simulink model "bee2_calib_corr"  (Simulink 7.1)
settings: Solver=ode45  StartTime=0.0  StopTime=100  RelTol=1e-3
undefined symbols (not defined in the file or in its folder): BEE2_usr
libraries: xbsIndex_r4 x2291, quant_xcorr_lib x1792, xps_library x56, xbsReplacements_r4 x32, casper_library_misc x2
(part 11 of 30)

== system bee2_calib_corr/slice6 ==
  in : Inport  OutDataType=sfix(16)  OutScaling=2^0
  Slice1 : xbsIndex_r4/Slice  nbits=2  boolean_output=off  mode=Upper Bit Location + Width  bit1=-2  base1=MSB of Input  bit0=0  base0=LSB of Input  dbl_ovrd=off  block_type=slice
      settings: infoedit="Extracts a given range of bits from each input sample and presents it at the output.  The output type is ordinarily unsigned with binary point at zero, but can be Boolean when the slice is one bit wide.<P><P>Hardware notes: In hardware this block costs nothing."  block_version=10.1.3
  Slice2 : xbsIndex_r4/Slice  nbits=2  boolean_output=off  mode=Upper Bit Location + Width  bit1=-6  base1=MSB of Input  bit0=0  base0=LSB of Input  dbl_ovrd=off  block_type=slice
      settings: infoedit="Extracts a given range of bits from each input sample and presents it at the output.  The output type is ordinarily unsigned with binary point at zero, but can be Boolean when the slice is one bit wide.<P><P>Hardware notes: In hardware this block costs nothing."  block_version=10.1.3
  Slice26 : xbsIndex_r4/Slice  nbits=2  boolean_output=off  mode=Upper Bit Location + Width  bit1=0  base1=MSB of Input  bit0=0  base0=LSB of Input  dbl_ovrd=off  block_type=slice
      settings: infoedit="Extracts a given range of bits from each input sample and presents it at the output.  The output type is ordinarily unsigned with binary point at zero, but can be Boolean when the slice is one bit wide.<P><P>Hardware notes: In hardware this block costs nothing."  block_version=10.1.3
  Slice3 : xbsIndex_r4/Slice  nbits=2  boolean_output=off  mode=Upper Bit Location + Width  bit1=-4  base1=MSB of Input  bit0=0  base0=LSB of Input  dbl_ovrd=off  block_type=slice
      settings: infoedit="Extracts a given range of bits from each input sample and presents it at the output.  The output type is ordinarily unsigned with binary point at zero, but can be Boolean when the slice is one bit wide.<P><P>Hardware notes: In hardware this block costs nothing."  block_version=10.1.3
  Slice4 : xbsIndex_r4/Slice  nbits=2  boolean_output=off  mode=Upper Bit Location + Width  bit1=-10  base1=MSB of Input  bit0=0  base0=LSB of Input  dbl_ovrd=off  block_type=slice
      settings: infoedit="Extracts a given range of bits from each input sample and presents it at the output.  The output type is ordinarily unsigned with binary point at zero, but can be Boolean when the slice is one bit wide.<P><P>Hardware notes: In hardware this block costs nothing."  block_version=10.1.3
  Slice5 : xbsIndex_r4/Slice  nbits=2  boolean_output=off  mode=Upper Bit Location + Width  bit1=-14  base1=MSB of Input  bit0=0  base0=LSB of Input  dbl_ovrd=off  block_type=slice
      settings: infoedit="Extracts a given range of bits from each input sample and presents it at the output.  The output type is ordinarily unsigned with binary point at zero, but can be Boolean when the slice is one bit wide.<P><P>Hardware notes: In hardware this block costs nothing."  block_version=10.1.3
  Slice6 : xbsIndex_r4/Slice  nbits=2  boolean_output=off  mode=Upper Bit Location + Width  bit1=-8  base1=MSB of Input  bit0=0  base0=LSB of Input  dbl_ovrd=off  block_type=slice
      settings: infoedit="Extracts a given range of bits from each input sample and presents it at the output.  The output type is ordinarily unsigned with binary point at zero, but can be Boolean when the slice is one bit wide.<P><P>Hardware notes: In hardware this block costs nothing."  block_version=10.1.3
  Slice7 : xbsIndex_r4/Slice  nbits=2  boolean_output=off  mode=Upper Bit Location + Width  bit1=-12  base1=MSB of Input  bit0=0  base0=LSB of Input  dbl_ovrd=off  block_type=slice
      settings: infoedit="Extracts a given range of bits from each input sample and presents it at the output.  The output type is ordinarily unsigned with binary point at zero, but can be Boolean when the slice is one bit wide.<P><P>Hardware notes: In hardware this block costs nothing."  block_version=10.1.3
  out0 : Outport  OutDataType=sfix(16)  OutScaling=2^0
  out1 : Outport  Port=2  OutDataType=sfix(16)  OutScaling=2^0
  out2 : Outport  Port=3  OutDataType=sfix(16)  OutScaling=2^0
  out3 : Outport  Port=4  OutDataType=sfix(16)  OutScaling=2^0
  out4 : Outport  Port=5  OutDataType=sfix(16)  OutScaling=2^0
  out5 : Outport  Port=6  OutDataType=sfix(16)  OutScaling=2^0
  out6 : Outport  Port=7  OutDataType=sfix(16)  OutScaling=2^0
  out7 : Outport  Port=8  OutDataType=sfix(16)  OutScaling=2^0
  wires:
  in/1 -> Slice26/1, Slice5/1, Slice7/1, Slice4/1, Slice6/1, Slice2/1, Slice3/1, Slice1/1
  Slice26/1 -> out0/1
  Slice1/1 -> out1/1
  Slice3/1 -> out2/1
  Slice2/1 -> out3/1
  Slice6/1 -> out4/1
  Slice4/1 -> out5/1
  Slice7/1 -> out6/1
  Slice5/1 -> out7/1

== system bee2_calib_corr/slice7 ==
  in : Inport  OutDataType=sfix(16)  OutScaling=2^0
  Slice1 : xbsIndex_r4/Slice  nbits=2  boolean_output=off  mode=Upper Bit Location + Width  bit1=-2  base1=MSB of Input  bit0=0  base0=LSB of Input  dbl_ovrd=off  block_type=slice
      settings: infoedit="Extracts a given range of bits from each input sample and presents it at the output.  The output type is ordinarily unsigned with binary point at zero, but can be Boolean when the slice is one bit wide.<P><P>Hardware notes: In hardware this block costs nothing."  block_version=10.1.3
  Slice2 : xbsIndex_r4/Slice  nbits=2  boolean_output=off  mode=Upper Bit Location + Width  bit1=-6  base1=MSB of Input  bit0=0  base0=LSB of Input  dbl_ovrd=off  block_type=slice
      settings: infoedit="Extracts a given range of bits from each input sample and presents it at the output.  The output type is ordinarily unsigned with binary point at zero, but can be Boolean when the slice is one bit wide.<P><P>Hardware notes: In hardware this block costs nothing."  block_version=10.1.3
  Slice26 : xbsIndex_r4/Slice  nbits=2  boolean_output=off  mode=Upper Bit Location + Width  bit1=0  base1=MSB of Input  bit0=0  base0=LSB of Input  dbl_ovrd=off  block_type=slice
      settings: infoedit="Extracts a given range of bits from each input sample and presents it at the output.  The output type is ordinarily unsigned with binary point at zero, but can be Boolean when the slice is one bit wide.<P><P>Hardware notes: In hardware this block costs nothing."  block_version=10.1.3
  Slice3 : xbsIndex_r4/Slice  nbits=2  boolean_output=off  mode=Upper Bit Location + Width  bit1=-4  base1=MSB of Input  bit0=0  base0=LSB of Input  dbl_ovrd=off  block_type=slice
      settings: infoedit="Extracts a given range of bits from each input sample and presents it at the output.  The output type is ordinarily unsigned with binary point at zero, but can be Boolean when the slice is one bit wide.<P><P>Hardware notes: In hardware this block costs nothing."  block_version=10.1.3
  Slice4 : xbsIndex_r4/Slice  nbits=2  boolean_output=off  mode=Upper Bit Location + Width  bit1=-10  base1=MSB of Input  bit0=0  base0=LSB of Input  dbl_ovrd=off  block_type=slice
      settings: infoedit="Extracts a given range of bits from each input sample and presents it at the output.  The output type is ordinarily unsigned with binary point at zero, but can be Boolean when the slice is one bit wide.<P><P>Hardware notes: In hardware this block costs nothing."  block_version=10.1.3
  Slice5 : xbsIndex_r4/Slice  nbits=2  boolean_output=off  mode=Upper Bit Location + Width  bit1=-14  base1=MSB of Input  bit0=0  base0=LSB of Input  dbl_ovrd=off  block_type=slice
      settings: infoedit="Extracts a given range of bits from each input sample and presents it at the output.  The output type is ordinarily unsigned with binary point at zero, but can be Boolean when the slice is one bit wide.<P><P>Hardware notes: In hardware this block costs nothing."  block_version=10.1.3
  Slice6 : xbsIndex_r4/Slice  nbits=2  boolean_output=off  mode=Upper Bit Location + Width  bit1=-8  base1=MSB of Input  bit0=0  base0=LSB of Input  dbl_ovrd=off  block_type=slice
      settings: infoedit="Extracts a given range of bits from each input sample and presents it at the output.  The output type is ordinarily unsigned with binary point at zero, but can be Boolean when the slice is one bit wide.<P><P>Hardware notes: In hardware this block costs nothing."  block_version=10.1.3
  Slice7 : xbsIndex_r4/Slice  nbits=2  boolean_output=off  mode=Upper Bit Location + Width  bit1=-12  base1=MSB of Input  bit0=0  base0=LSB of Input  dbl_ovrd=off  block_type=slice
      settings: infoedit="Extracts a given range of bits from each input sample and presents it at the output.  The output type is ordinarily unsigned with binary point at zero, but can be Boolean when the slice is one bit wide.<P><P>Hardware notes: In hardware this block costs nothing."  block_version=10.1.3
  out0 : Outport  OutDataType=sfix(16)  OutScaling=2^0
  out1 : Outport  Port=2  OutDataType=sfix(16)  OutScaling=2^0
  out2 : Outport  Port=3  OutDataType=sfix(16)  OutScaling=2^0
  out3 : Outport  Port=4  OutDataType=sfix(16)  OutScaling=2^0
  out4 : Outport  Port=5  OutDataType=sfix(16)  OutScaling=2^0
  out5 : Outport  Port=6  OutDataType=sfix(16)  OutScaling=2^0
  out6 : Outport  Port=7  OutDataType=sfix(16)  OutScaling=2^0
  out7 : Outport  Port=8  OutDataType=sfix(16)  OutScaling=2^0
  wires:
  Slice5/1 -> out7/1
  Slice7/1 -> out6/1
  Slice4/1 -> out5/1
  Slice6/1 -> out4/1
  Slice2/1 -> out3/1
  Slice3/1 -> out2/1
  Slice1/1 -> out1/1
  Slice26/1 -> out0/1
  in/1 -> Slice1/1, Slice3/1, Slice2/1, Slice6/1, Slice4/1, Slice7/1, Slice5/1, Slice26/1

== system bee2_calib_corr/slice8 ==
  in : Inport  OutDataType=sfix(16)  OutScaling=2^0
  Slice1 : xbsIndex_r4/Slice  nbits=2  boolean_output=off  mode=Upper Bit Location + Width  bit1=-2  base1=MSB of Input  bit0=0  base0=LSB of Input  dbl_ovrd=off  block_type=slice
      settings: infoedit="Extracts a given range of bits from each input sample and presents it at the output.  The output type is ordinarily unsigned with binary point at zero, but can be Boolean when the slice is one bit wide.<P><P>Hardware notes: In hardware this block costs nothing."  block_version=10.1.3
  Slice2 : xbsIndex_r4/Slice  nbits=2  boolean_output=off  mode=Upper Bit Location + Width  bit1=-6  base1=MSB of Input  bit0=0  base0=LSB of Input  dbl_ovrd=off  block_type=slice
      settings: infoedit="Extracts a given range of bits from each input sample and presents it at the output.  The output type is ordinarily unsigned with binary point at zero, but can be Boolean when the slice is one bit wide.<P><P>Hardware notes: In hardware this block costs nothing."  block_version=10.1.3
  Slice26 : xbsIndex_r4/Slice  nbits=2  boolean_output=off  mode=Upper Bit Location + Width  bit1=0  base1=MSB of Input  bit0=0  base0=LSB of Input  dbl_ovrd=off  block_type=slice
      settings: infoedit="Extracts a given range of bits from each input sample and presents it at the output.  The output type is ordinarily unsigned with binary point at zero, but can be Boolean when the slice is one bit wide.<P><P>Hardware notes: In hardware this block costs nothing."  block_version=10.1.3
  Slice3 : xbsIndex_r4/Slice  nbits=2  boolean_output=off  mode=Upper Bit Location + Width  bit1=-4  base1=MSB of Input  bit0=0  base0=LSB of Input  dbl_ovrd=off  block_type=slice
      settings: infoedit="Extracts a given range of bits from each input sample and presents it at the output.  The output type is ordinarily unsigned with binary point at zero, but can be Boolean when the slice is one bit wide.<P><P>Hardware notes: In hardware this block costs nothing."  block_version=10.1.3
  Slice4 : xbsIndex_r4/Slice  nbits=2  boolean_output=off  mode=Upper Bit Location + Width  bit1=-10  base1=MSB of Input  bit0=0  base0=LSB of Input  dbl_ovrd=off  block_type=slice
      settings: infoedit="Extracts a given range of bits from each input sample and presents it at the output.  The output type is ordinarily unsigned with binary point at zero, but can be Boolean when the slice is one bit wide.<P><P>Hardware notes: In hardware this block costs nothing."  block_version=10.1.3
  Slice5 : xbsIndex_r4/Slice  nbits=2  boolean_output=off  mode=Upper Bit Location + Width  bit1=-14  base1=MSB of Input  bit0=0  base0=LSB of Input  dbl_ovrd=off  block_type=slice
      settings: infoedit="Extracts a given range of bits from each input sample and presents it at the output.  The output type is ordinarily unsigned with binary point at zero, but can be Boolean when the slice is one bit wide.<P><P>Hardware notes: In hardware this block costs nothing."  block_version=10.1.3
  Slice6 : xbsIndex_r4/Slice  nbits=2  boolean_output=off  mode=Upper Bit Location + Width  bit1=-8  base1=MSB of Input  bit0=0  base0=LSB of Input  dbl_ovrd=off  block_type=slice
      settings: infoedit="Extracts a given range of bits from each input sample and presents it at the output.  The output type is ordinarily unsigned with binary point at zero, but can be Boolean when the slice is one bit wide.<P><P>Hardware notes: In hardware this block costs nothing."  block_version=10.1.3
  Slice7 : xbsIndex_r4/Slice  nbits=2  boolean_output=off  mode=Upper Bit Location + Width  bit1=-12  base1=MSB of Input  bit0=0  base0=LSB of Input  dbl_ovrd=off  block_type=slice
      settings: infoedit="Extracts a given range of bits from each input sample and presents it at the output.  The output type is ordinarily unsigned with binary point at zero, but can be Boolean when the slice is one bit wide.<P><P>Hardware notes: In hardware this block costs nothing."  block_version=10.1.3
  out0 : Outport  OutDataType=sfix(16)  OutScaling=2^0
  out1 : Outport  Port=2  OutDataType=sfix(16)  OutScaling=2^0
  out2 : Outport  Port=3  OutDataType=sfix(16)  OutScaling=2^0
  out3 : Outport  Port=4  OutDataType=sfix(16)  OutScaling=2^0
  out4 : Outport  Port=5  OutDataType=sfix(16)  OutScaling=2^0
  out5 : Outport  Port=6  OutDataType=sfix(16)  OutScaling=2^0
  out6 : Outport  Port=7  OutDataType=sfix(16)  OutScaling=2^0
  out7 : Outport  Port=8  OutDataType=sfix(16)  OutScaling=2^0
  wires:
  in/1 -> Slice26/1, Slice5/1, Slice7/1, Slice4/1, Slice6/1, Slice2/1, Slice3/1, Slice1/1
  Slice26/1 -> out0/1
  Slice1/1 -> out1/1
  Slice3/1 -> out2/1
  Slice2/1 -> out3/1
  Slice6/1 -> out4/1
  Slice4/1 -> out5/1
  Slice7/1 -> out6/1
  Slice5/1 -> out7/1

== system bee2_calib_corr/slice9 ==
  in : Inport  OutDataType=sfix(16)  OutScaling=2^0
  Slice1 : xbsIndex_r4/Slice  nbits=2  boolean_output=off  mode=Upper Bit Location + Width  bit1=-2  base1=MSB of Input  bit0=0  base0=LSB of Input  dbl_ovrd=off  block_type=slice
      settings: infoedit="Extracts a given range of bits from each input sample and presents it at the output.  The output type is ordinarily unsigned with binary point at zero, but can be Boolean when the slice is one bit wide.<P><P>Hardware notes: In hardware this block costs nothing."  block_version=10.1.3
  Slice2 : xbsIndex_r4/Slice  nbits=2  boolean_output=off  mode=Upper Bit Location + Width  bit1=-6  base1=MSB of Input  bit0=0  base0=LSB of Input  dbl_ovrd=off  block_type=slice
      settings: infoedit="Extracts a given range of bits from each input sample and presents it at the output.  The output type is ordinarily unsigned with binary point at zero, but can be Boolean when the slice is one bit wide.<P><P>Hardware notes: In hardware this block costs nothing."  block_version=10.1.3
  Slice26 : xbsIndex_r4/Slice  nbits=2  boolean_output=off  mode=Upper Bit Location + Width  bit1=0  base1=MSB of Input  bit0=0  base0=LSB of Input  dbl_ovrd=off  block_type=slice
      settings: infoedit="Extracts a given range of bits from each input sample and presents it at the output.  The output type is ordinarily unsigned with binary point at zero, but can be Boolean when the slice is one bit wide.<P><P>Hardware notes: In hardware this block costs nothing."  block_version=10.1.3
  Slice3 : xbsIndex_r4/Slice  nbits=2  boolean_output=off  mode=Upper Bit Location + Width  bit1=-4  base1=MSB of Input  bit0=0  base0=LSB of Input  dbl_ovrd=off  block_type=slice
      settings: infoedit="Extracts a given range of bits from each input sample and presents it at the output.  The output type is ordinarily unsigned with binary point at zero, but can be Boolean when the slice is one bit wide.<P><P>Hardware notes: In hardware this block costs nothing."  block_version=10.1.3
  Slice4 : xbsIndex_r4/Slice  nbits=2  boolean_output=off  mode=Upper Bit Location + Width  bit1=-10  base1=MSB of Input  bit0=0  base0=LSB of Input  dbl_ovrd=off  block_type=slice
      settings: infoedit="Extracts a given range of bits from each input sample and presents it at the output.  The output type is ordinarily unsigned with binary point at zero, but can be Boolean when the slice is one bit wide.<P><P>Hardware notes: In hardware this block costs nothing."  block_version=10.1.3
  Slice5 : xbsIndex_r4/Slice  nbits=2  boolean_output=off  mode=Upper Bit Location + Width  bit1=-14  base1=MSB of Input  bit0=0  base0=LSB of Input  dbl_ovrd=off  block_type=slice
      settings: infoedit="Extracts a given range of bits from each input sample and presents it at the output.  The output type is ordinarily unsigned with binary point at zero, but can be Boolean when the slice is one bit wide.<P><P>Hardware notes: In hardware this block costs nothing."  block_version=10.1.3
  Slice6 : xbsIndex_r4/Slice  nbits=2  boolean_output=off  mode=Upper Bit Location + Width  bit1=-8  base1=MSB of Input  bit0=0  base0=LSB of Input  dbl_ovrd=off  block_type=slice
      settings: infoedit="Extracts a given range of bits from each input sample and presents it at the output.  The output type is ordinarily unsigned with binary point at zero, but can be Boolean when the slice is one bit wide.<P><P>Hardware notes: In hardware this block costs nothing."  block_version=10.1.3
  Slice7 : xbsIndex_r4/Slice  nbits=2  boolean_output=off  mode=Upper Bit Location + Width  bit1=-12  base1=MSB of Input  bit0=0  base0=LSB of Input  dbl_ovrd=off  block_type=slice
      settings: infoedit="Extracts a given range of bits from each input sample and presents it at the output.  The output type is ordinarily unsigned with binary point at zero, but can be Boolean when the slice is one bit wide.<P><P>Hardware notes: In hardware this block costs nothing."  block_version=10.1.3
  out0 : Outport  OutDataType=sfix(16)  OutScaling=2^0
  out1 : Outport  Port=2  OutDataType=sfix(16)  OutScaling=2^0
  out2 : Outport  Port=3  OutDataType=sfix(16)  OutScaling=2^0
  out3 : Outport  Port=4  OutDataType=sfix(16)  OutScaling=2^0
  out4 : Outport  Port=5  OutDataType=sfix(16)  OutScaling=2^0
  out5 : Outport  Port=6  OutDataType=sfix(16)  OutScaling=2^0
  out6 : Outport  Port=7  OutDataType=sfix(16)  OutScaling=2^0
  out7 : Outport  Port=8  OutDataType=sfix(16)  OutScaling=2^0
  wires:
  Slice5/1 -> out7/1
  Slice7/1 -> out6/1
  Slice4/1 -> out5/1
  Slice6/1 -> out4/1
  Slice2/1 -> out3/1
  Slice3/1 -> out2/1
  Slice1/1 -> out1/1
  Slice26/1 -> out0/1
  in/1 -> Slice1/1, Slice3/1, Slice2/1, Slice6/1, Slice4/1, Slice7/1, Slice5/1, Slice26/1

== system bee2_calib_corr/valid ==
  ld0 : Inport  OutDataType=sfix(16)  OutScaling=2^0
  ld1 : Inport  Port=2  OutDataType=sfix(16)  OutScaling=2^0
  align : Inport  Port=3  OutDataType=sfix(16)  OutScaling=2^0
  Constant1 : xbsIndex_r4/Constant  arith_type=Boolean  const=1  n_bits=1  bin_pt=0  explicit_period=on  period=1  equ=P=C  opselect=C  inp2=PCIN>>17  opr=+  inp1=P  carry=CIN  dbl_ovrd=off  block_type=constant
  Constant3 : xbsIndex_r4/Constant  arith_type=Boolean  const=1  n_bits=1  bin_pt=0  explicit_period=on  period=1  equ=P=C  opselect=C  inp2=PCIN>>17  opr=+  inp1=P  carry=CIN  dbl_ovrd=off  block_type=constant
  Convert1 : xbsIndex_r4/Convert  arith_type=Boolean  n_bits=8  bin_pt=6  quantization=Truncate  overflow=Wrap  latency=0  dbl_ovrd=off  pipeline=off  block_type=convert
      settings: xl_area=[0, 0, 0, 0, 0, 0, 0]  block_version=10.1.3
  Convert3 : xbsIndex_r4/Convert  arith_type=Boolean  n_bits=8  bin_pt=6  quantization=Truncate  overflow=Wrap  latency=0  dbl_ovrd=off  pipeline=off  block_type=convert
      settings: xl_area=[0, 0, 0, 0, 0, 0, 0]  block_version=10.1.3
  Expression : xbsIndex_r4/Expression  expression=a & b & c  align_bp=on  en=off  latency=0  precision=Full  arith_type=Unsigned  n_bits=8  bin_pt=2  dbl_ovrd=off  block_type=expr
      settings: xl_area=[0, 0, 0, 0, 0, 0, 0]  block_version=10.1.3
  Inverter1 : xbsIndex_r4/Inverter  en=off  latency=0  dbl_ovrd=off  block_type=inv
      settings: xl_area=[0, 0, 0, 0, 0, 0, 0]
  Inverter2 : xbsIndex_r4/Inverter  en=off  latency=0  dbl_ovrd=off  block_type=inv
      settings: xl_area=[0, 0, 0, 0, 0, 0, 0]
  Simulation Multiplexer1 : xbsIndex_r4/Simulation Multiplexer  sim_sel=1  hw_sel=2  block_type=simmux
      settings: infoedit="Distinguish input subsystems as \"simulation only\" and \"generation only\".  The input specified For Simulation will be used during Simulink simulation.  The input specified For Generation will be used during code generation.  This block will typically be used on the output of a HW Co-Simulation, Black Box, or ModelSim subsystem.<P><P>Hardware Notes: This block costs nothing."  block_version=10.1.3
  Simulation Multiplexer3 : xbsIndex_r4/Simulation Multiplexer  sim_sel=1  hw_sel=2  block_type=simmux
      settings: infoedit="Distinguish input subsystems as \"simulation only\" and \"generation only\".  The input specified For Simulation will be used during Simulink simulation.  The input specified For Generation will be used during code generation.  This block will typically be used on the output of a HW Co-Simulation, Black Box, or ModelSim subsystem.<P><P>Hardware Notes: This block costs nothing."  block_version=10.1.3
  get : Outport  OutDataType=sfix(16)  OutScaling=2^0
  wires:
  Convert1/1 -> Inverter1/1
  Convert3/1 -> Inverter2/1
  ld0/1 -> Convert1/1
  ld1/1 -> Convert3/1
  align/1 -> Expression/3
  Expression/1 -> get/1
  Constant1/1 -> Simulation Multiplexer1/1
  Inverter1/1 -> Simulation Multiplexer1/2
  Constant3/1 -> Simulation Multiplexer3/1
  Inverter2/1 -> Simulation Multiplexer3/2
  Simulation Multiplexer3/1 -> Expression/2
  Simulation Multiplexer1/1 -> Expression/1

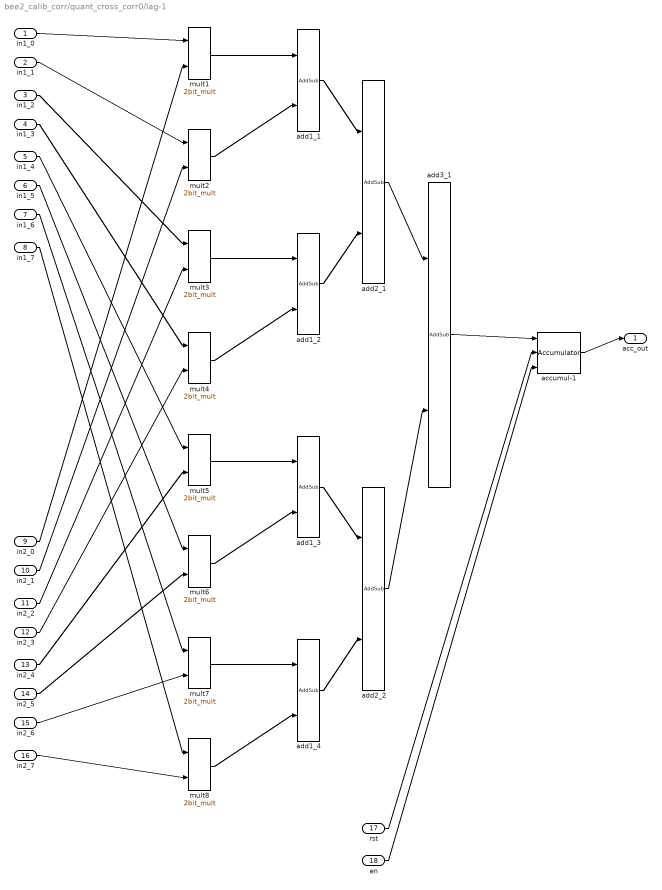
== system bee2_calib_corr/quant_cross_corr0/lag-1 ==
  in1_0 : Inport  OutDataType=sfix(16)  OutScaling=2^0
  in1_1 : Inport  Port=2  OutDataType=sfix(16)  OutScaling=2^0
  in1_2 : Inport  Port=3  OutDataType=sfix(16)  OutScaling=2^0
  in1_3 : Inport  Port=4  OutDataType=sfix(16)  OutScaling=2^0
  in1_4 : Inport  Port=5  OutDataType=sfix(16)  OutScaling=2^0
  in1_5 : Inport  Port=6  OutDataType=sfix(16)  OutScaling=2^0
  in1_6 : Inport  Port=7  OutDataType=sfix(16)  OutScaling=2^0
  in1_7 : Inport  Port=8  OutDataType=sfix(16)  OutScaling=2^0
  in2_0 : Inport  Port=9  OutDataType=sfix(16)  OutScaling=2^0
  in2_1 : Inport  Port=10  OutDataType=sfix(16)  OutScaling=2^0
  in2_2 : Inport  Port=11  OutDataType=sfix(16)  OutScaling=2^0
  in2_3 : Inport  Port=12  OutDataType=sfix(16)  OutScaling=2^0
  in2_4 : Inport  Port=13  OutDataType=sfix(16)  OutScaling=2^0
  in2_5 : Inport  Port=14  OutDataType=sfix(16)  OutScaling=2^0
  in2_6 : Inport  Port=15  OutDataType=sfix(16)  OutScaling=2^0
  in2_7 : Inport  Port=16  OutDataType=sfix(16)  OutScaling=2^0
  rst : Inport  Port=17  OutDataType=sfix(16)  OutScaling=2^0
  en : Inport  Port=18  OutDataType=sfix(16)  OutScaling=2^0
  accumul-1 : xbsIndex_r4/Accumulator  operation=Add  n_bits=32  overflow=Saturate  scale=1  rst=on  hasbypass=off  en=on  dbl_ovrd=off  use_behavioral_HDL=on  implementation=Fabric  block_type=accum
      settings: infoedit="Adder or subtractor-based accumulator.   Output type and binary point position match the input.<P><P>Hardware notes: When \"Reinitialize with input 'b' on reset\" is selected, the accumulator is forced to run at the system rate even if the input 'b' is running at a slower rate."  xl_area=[0, 0, 0, 0, 0, 0, 0]  block_version=10.1.3
  add1_{1..4} (4 alike) : xbsIndex_r4/AddSub  mode=Addition  use_carryin=off  use_carryout=off  en=off  latency=1  precision=Full  arith_type=Unsigned  n_bits=8  bin_pt=2  quantization=Truncate  overflow=Wrap  dbl_ovrd=off  use_behavioral_HDL=on  pipelined=on  use_rpm=on  hw_selection=Fabric  block_type=addsub
      settings: xl_area=[0, 0, 0, 0, 0, 0, 0]  block_version=10.1.3
  add2_1 : xbsIndex_r4/AddSub  mode=Addition  use_carryin=off  use_carryout=off  en=off  latency=1  precision=Full  arith_type=Unsigned  n_bits=8  bin_pt=2  quantization=Truncate  overflow=Wrap  dbl_ovrd=off  use_behavioral_HDL=on  pipelined=on  use_rpm=on  hw_selection=Fabric  block_type=addsub
      settings: xl_area=[0, 0, 0, 0, 0, 0, 0]  block_version=10.1.3
  add2_2 : xbsIndex_r4/AddSub  mode=Addition  use_carryin=off  use_carryout=off  en=off  latency=1  precision=Full  arith_type=Unsigned  n_bits=8  bin_pt=2  quantization=Truncate  overflow=Wrap  dbl_ovrd=off  use_behavioral_HDL=on  pipelined=on  use_rpm=on  hw_selection=Fabric  block_type=addsub
      settings: xl_area=[0, 0, 0, 0, 0, 0, 0]  block_version=10.1.3
  add3_1 : xbsIndex_r4/AddSub  mode=Addition  use_carryin=off  use_carryout=off  en=off  latency=1  precision=Full  arith_type=Unsigned  n_bits=8  bin_pt=2  quantization=Truncate  overflow=Wrap  dbl_ovrd=off  use_behavioral_HDL=on  pipelined=on  use_rpm=on  hw_selection=Fabric  block_type=addsub
      settings: xl_area=[0, 0, 0, 0, 0, 0, 0]  block_version=10.1.3
  mult{1..8} (8 alike) : quant_xcorr_lib/2bit_mult  SystemSampleTime=-1
  acc_out : Outport  OutDataType=sfix(16)  OutScaling=2^0
  wires:
  in1_0/1 -> mult1/1
  in2_0/1 -> mult1/2
  in1_1/1 -> mult2/1
  in2_1/1 -> mult2/2
  in1_2/1 -> mult3/1
  in2_2/1 -> mult3/2
  in1_3/1 -> mult4/1
  in2_3/1 -> mult4/2
  in1_4/1 -> mult5/1
  in2_4/1 -> mult5/2
  in1_5/1 -> mult6/1
  in2_5/1 -> mult6/2
  in1_6/1 -> mult7/1
  in2_6/1 -> mult7/2
  in1_7/1 -> mult8/1
  in2_7/1 -> mult8/2
  mult1/1 -> add1_1/1
  mult2/1 -> add1_1/2
  mult3/1 -> add1_2/1
  mult4/1 -> add1_2/2
  mult5/1 -> add1_3/1
  mult6/1 -> add1_3/2
  mult7/1 -> add1_4/1
  mult8/1 -> add1_4/2
  add1_1/1 -> add2_1/1
  add1_2/1 -> add2_1/2
  add1_3/1 -> add2_2/1
  add1_4/1 -> add2_2/2
  add2_1/1 -> add3_1/1
  add2_2/1 -> add3_1/2
  add3_1/1 -> accumul-1/1
  accumul-1/1 -> acc_out/1
  rst/1 -> accumul-1/2
  en/1 -> accumul-1/3

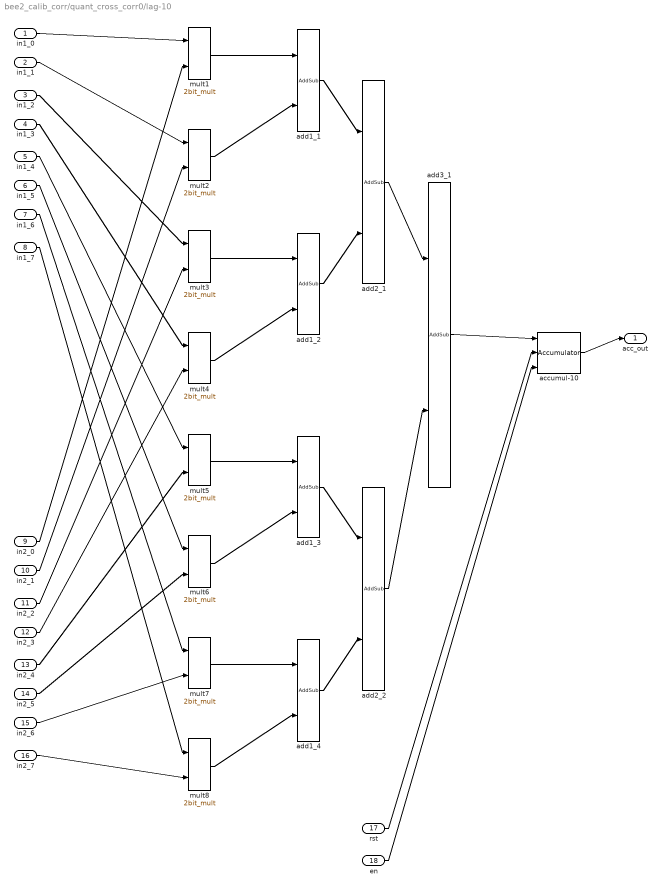
== system bee2_calib_corr/quant_cross_corr0/lag-10 ==
  in1_0 : Inport  OutDataType=sfix(16)  OutScaling=2^0
  in1_1 : Inport  Port=2  OutDataType=sfix(16)  OutScaling=2^0
  in1_2 : Inport  Port=3  OutDataType=sfix(16)  OutScaling=2^0
  in1_3 : Inport  Port=4  OutDataType=sfix(16)  OutScaling=2^0
  in1_4 : Inport  Port=5  OutDataType=sfix(16)  OutScaling=2^0
  in1_5 : Inport  Port=6  OutDataType=sfix(16)  OutScaling=2^0
  in1_6 : Inport  Port=7  OutDataType=sfix(16)  OutScaling=2^0
  in1_7 : Inport  Port=8  OutDataType=sfix(16)  OutScaling=2^0
  in2_0 : Inport  Port=9  OutDataType=sfix(16)  OutScaling=2^0
  in2_1 : Inport  Port=10  OutDataType=sfix(16)  OutScaling=2^0
  in2_2 : Inport  Port=11  OutDataType=sfix(16)  OutScaling=2^0
  in2_3 : Inport  Port=12  OutDataType=sfix(16)  OutScaling=2^0
  in2_4 : Inport  Port=13  OutDataType=sfix(16)  OutScaling=2^0
  in2_5 : Inport  Port=14  OutDataType=sfix(16)  OutScaling=2^0
  in2_6 : Inport  Port=15  OutDataType=sfix(16)  OutScaling=2^0
  in2_7 : Inport  Port=16  OutDataType=sfix(16)  OutScaling=2^0
  rst : Inport  Port=17  OutDataType=sfix(16)  OutScaling=2^0
  en : Inport  Port=18  OutDataType=sfix(16)  OutScaling=2^0
  accumul-10 : xbsIndex_r4/Accumulator  operation=Add  n_bits=32  overflow=Saturate  scale=1  rst=on  hasbypass=off  en=on  dbl_ovrd=off  use_behavioral_HDL=on  implementation=Fabric  block_type=accum
      settings: infoedit="Adder or subtractor-based accumulator.   Output type and binary point position match the input.<P><P>Hardware notes: When \"Reinitialize with input 'b' on reset\" is selected, the accumulator is forced to run at the system rate even if the input 'b' is running at a slower rate."  xl_area=[0, 0, 0, 0, 0, 0, 0]  block_version=10.1.3
  add1_{1..4} (4 alike) : xbsIndex_r4/AddSub  mode=Addition  use_carryin=off  use_carryout=off  en=off  latency=1  precision=Full  arith_type=Unsigned  n_bits=8  bin_pt=2  quantization=Truncate  overflow=Wrap  dbl_ovrd=off  use_behavioral_HDL=on  pipelined=on  use_rpm=on  hw_selection=Fabric  block_type=addsub
      settings: xl_area=[0, 0, 0, 0, 0, 0, 0]  block_version=10.1.3
  add2_1 : xbsIndex_r4/AddSub  mode=Addition  use_carryin=off  use_carryout=off  en=off  latency=1  precision=Full  arith_type=Unsigned  n_bits=8  bin_pt=2  quantization=Truncate  overflow=Wrap  dbl_ovrd=off  use_behavioral_HDL=on  pipelined=on  use_rpm=on  hw_selection=Fabric  block_type=addsub
      settings: xl_area=[0, 0, 0, 0, 0, 0, 0]  block_version=10.1.3
  add2_2 : xbsIndex_r4/AddSub  mode=Addition  use_carryin=off  use_carryout=off  en=off  latency=1  precision=Full  arith_type=Unsigned  n_bits=8  bin_pt=2  quantization=Truncate  overflow=Wrap  dbl_ovrd=off  use_behavioral_HDL=on  pipelined=on  use_rpm=on  hw_selection=Fabric  block_type=addsub
      settings: xl_area=[0, 0, 0, 0, 0, 0, 0]  block_version=10.1.3
  add3_1 : xbsIndex_r4/AddSub  mode=Addition  use_carryin=off  use_carryout=off  en=off  latency=1  precision=Full  arith_type=Unsigned  n_bits=8  bin_pt=2  quantization=Truncate  overflow=Wrap  dbl_ovrd=off  use_behavioral_HDL=on  pipelined=on  use_rpm=on  hw_selection=Fabric  block_type=addsub
      settings: xl_area=[0, 0, 0, 0, 0, 0, 0]  block_version=10.1.3
  mult{1..8} (8 alike) : quant_xcorr_lib/2bit_mult  SystemSampleTime=-1
  acc_out : Outport  OutDataType=sfix(16)  OutScaling=2^0
  wires:
  in1_0/1 -> mult1/1
  in2_0/1 -> mult1/2
  in1_1/1 -> mult2/1
  in2_1/1 -> mult2/2
  in1_2/1 -> mult3/1
  in2_2/1 -> mult3/2
  in1_3/1 -> mult4/1
  in2_3/1 -> mult4/2
  in1_4/1 -> mult5/1
  in2_4/1 -> mult5/2
  in1_5/1 -> mult6/1
  in2_5/1 -> mult6/2
  in1_6/1 -> mult7/1
  in2_6/1 -> mult7/2
  in1_7/1 -> mult8/1
  in2_7/1 -> mult8/2
  mult1/1 -> add1_1/1
  mult2/1 -> add1_1/2
  mult3/1 -> add1_2/1
  mult4/1 -> add1_2/2
  mult5/1 -> add1_3/1
  mult6/1 -> add1_3/2
  mult7/1 -> add1_4/1
  mult8/1 -> add1_4/2
  add1_1/1 -> add2_1/1
  add1_2/1 -> add2_1/2
  add1_3/1 -> add2_2/1
  add1_4/1 -> add2_2/2
  add2_1/1 -> add3_1/1
  add2_2/1 -> add3_1/2
  add3_1/1 -> accumul-10/1
  accumul-10/1 -> acc_out/1
  rst/1 -> accumul-10/2
  en/1 -> accumul-10/3

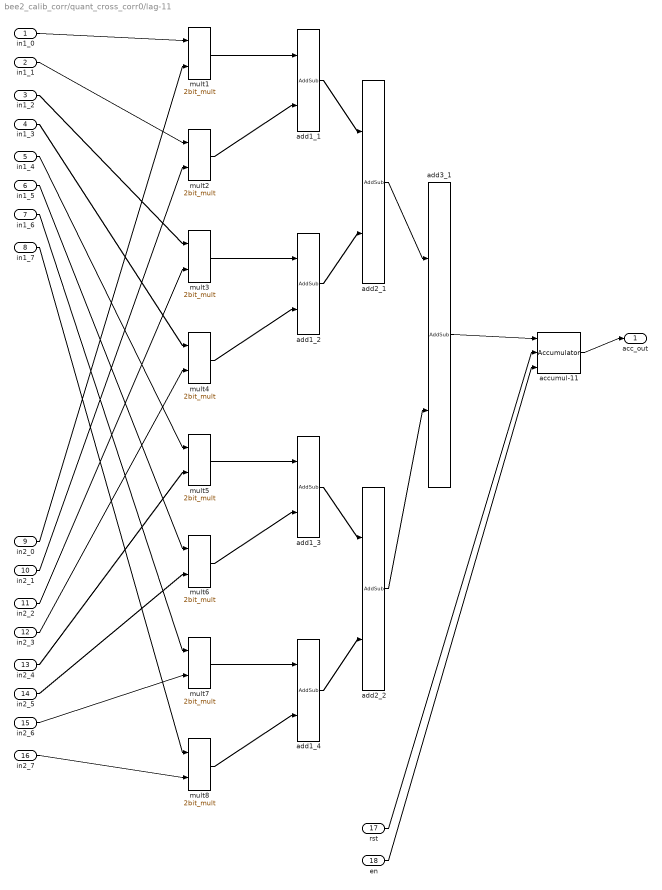
== system bee2_calib_corr/quant_cross_corr0/lag-11 ==
  in1_0 : Inport  OutDataType=sfix(16)  OutScaling=2^0
  in1_1 : Inport  Port=2  OutDataType=sfix(16)  OutScaling=2^0
  in1_2 : Inport  Port=3  OutDataType=sfix(16)  OutScaling=2^0
  in1_3 : Inport  Port=4  OutDataType=sfix(16)  OutScaling=2^0
  in1_4 : Inport  Port=5  OutDataType=sfix(16)  OutScaling=2^0
  in1_5 : Inport  Port=6  OutDataType=sfix(16)  OutScaling=2^0
  in1_6 : Inport  Port=7  OutDataType=sfix(16)  OutScaling=2^0
  in1_7 : Inport  Port=8  OutDataType=sfix(16)  OutScaling=2^0
  in2_0 : Inport  Port=9  OutDataType=sfix(16)  OutScaling=2^0
  in2_1 : Inport  Port=10  OutDataType=sfix(16)  OutScaling=2^0
  in2_2 : Inport  Port=11  OutDataType=sfix(16)  OutScaling=2^0
  in2_3 : Inport  Port=12  OutDataType=sfix(16)  OutScaling=2^0
  in2_4 : Inport  Port=13  OutDataType=sfix(16)  OutScaling=2^0
  in2_5 : Inport  Port=14  OutDataType=sfix(16)  OutScaling=2^0
  in2_6 : Inport  Port=15  OutDataType=sfix(16)  OutScaling=2^0
  in2_7 : Inport  Port=16  OutDataType=sfix(16)  OutScaling=2^0
  rst : Inport  Port=17  OutDataType=sfix(16)  OutScaling=2^0
  en : Inport  Port=18  OutDataType=sfix(16)  OutScaling=2^0
  accumul-11 : xbsIndex_r4/Accumulator  operation=Add  n_bits=32  overflow=Saturate  scale=1  rst=on  hasbypass=off  en=on  dbl_ovrd=off  use_behavioral_HDL=on  implementation=Fabric  block_type=accum
      settings: infoedit="Adder or subtractor-based accumulator.   Output type and binary point position match the input.<P><P>Hardware notes: When \"Reinitialize with input 'b' on reset\" is selected, the accumulator is forced to run at the system rate even if the input 'b' is running at a slower rate."  xl_area=[0, 0, 0, 0, 0, 0, 0]  block_version=10.1.3
  add1_{1..4} (4 alike) : xbsIndex_r4/AddSub  mode=Addition  use_carryin=off  use_carryout=off  en=off  latency=1  precision=Full  arith_type=Unsigned  n_bits=8  bin_pt=2  quantization=Truncate  overflow=Wrap  dbl_ovrd=off  use_behavioral_HDL=on  pipelined=on  use_rpm=on  hw_selection=Fabric  block_type=addsub
      settings: xl_area=[0, 0, 0, 0, 0, 0, 0]  block_version=10.1.3
  add2_1 : xbsIndex_r4/AddSub  mode=Addition  use_carryin=off  use_carryout=off  en=off  latency=1  precision=Full  arith_type=Unsigned  n_bits=8  bin_pt=2  quantization=Truncate  overflow=Wrap  dbl_ovrd=off  use_behavioral_HDL=on  pipelined=on  use_rpm=on  hw_selection=Fabric  block_type=addsub
      settings: xl_area=[0, 0, 0, 0, 0, 0, 0]  block_version=10.1.3
  add2_2 : xbsIndex_r4/AddSub  mode=Addition  use_carryin=off  use_carryout=off  en=off  latency=1  precision=Full  arith_type=Unsigned  n_bits=8  bin_pt=2  quantization=Truncate  overflow=Wrap  dbl_ovrd=off  use_behavioral_HDL=on  pipelined=on  use_rpm=on  hw_selection=Fabric  block_type=addsub
      settings: xl_area=[0, 0, 0, 0, 0, 0, 0]  block_version=10.1.3
  add3_1 : xbsIndex_r4/AddSub  mode=Addition  use_carryin=off  use_carryout=off  en=off  latency=1  precision=Full  arith_type=Unsigned  n_bits=8  bin_pt=2  quantization=Truncate  overflow=Wrap  dbl_ovrd=off  use_behavioral_HDL=on  pipelined=on  use_rpm=on  hw_selection=Fabric  block_type=addsub
      settings: xl_area=[0, 0, 0, 0, 0, 0, 0]  block_version=10.1.3
  mult{1..8} (8 alike) : quant_xcorr_lib/2bit_mult  SystemSampleTime=-1
  acc_out : Outport  OutDataType=sfix(16)  OutScaling=2^0
  wires:
  in1_0/1 -> mult1/1
  in2_0/1 -> mult1/2
  in1_1/1 -> mult2/1
  in2_1/1 -> mult2/2
  in1_2/1 -> mult3/1
  in2_2/1 -> mult3/2
  in1_3/1 -> mult4/1
  in2_3/1 -> mult4/2
  in1_4/1 -> mult5/1
  in2_4/1 -> mult5/2
  in1_5/1 -> mult6/1
  in2_5/1 -> mult6/2
  in1_6/1 -> mult7/1
  in2_6/1 -> mult7/2
  in1_7/1 -> mult8/1
  in2_7/1 -> mult8/2
  mult1/1 -> add1_1/1
  mult2/1 -> add1_1/2
  mult3/1 -> add1_2/1
  mult4/1 -> add1_2/2
  mult5/1 -> add1_3/1
  mult6/1 -> add1_3/2
  mult7/1 -> add1_4/1
  mult8/1 -> add1_4/2
  add1_1/1 -> add2_1/1
  add1_2/1 -> add2_1/2
  add1_3/1 -> add2_2/1
  add1_4/1 -> add2_2/2
  add2_1/1 -> add3_1/1
  add2_2/1 -> add3_1/2
  add3_1/1 -> accumul-11/1
  accumul-11/1 -> acc_out/1
  rst/1 -> accumul-11/2
  en/1 -> accumul-11/3

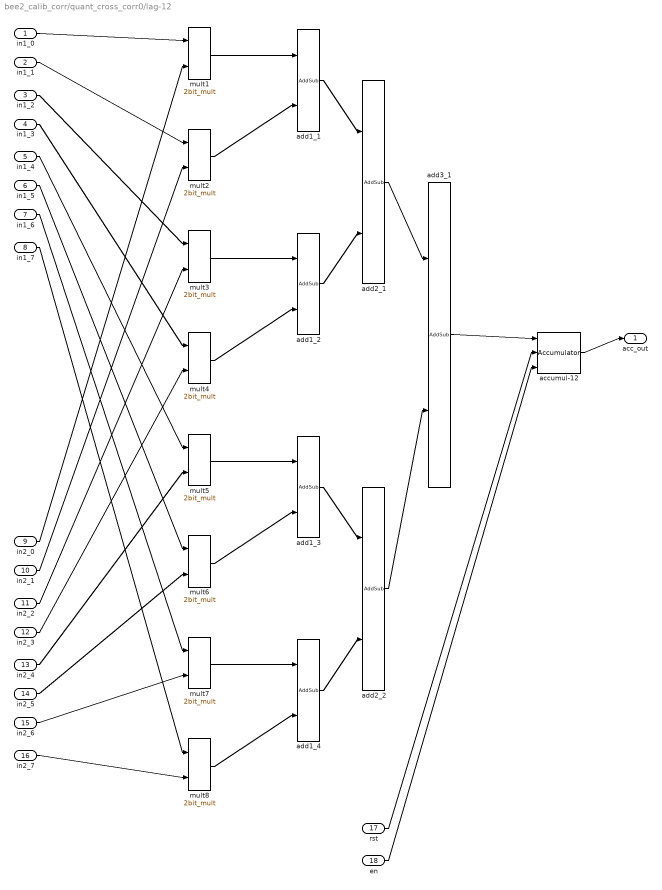
== system bee2_calib_corr/quant_cross_corr0/lag-12 ==
  in1_0 : Inport  OutDataType=sfix(16)  OutScaling=2^0
  in1_1 : Inport  Port=2  OutDataType=sfix(16)  OutScaling=2^0
  in1_2 : Inport  Port=3  OutDataType=sfix(16)  OutScaling=2^0
  in1_3 : Inport  Port=4  OutDataType=sfix(16)  OutScaling=2^0
  in1_4 : Inport  Port=5  OutDataType=sfix(16)  OutScaling=2^0
  in1_5 : Inport  Port=6  OutDataType=sfix(16)  OutScaling=2^0
  in1_6 : Inport  Port=7  OutDataType=sfix(16)  OutScaling=2^0
  in1_7 : Inport  Port=8  OutDataType=sfix(16)  OutScaling=2^0
  in2_0 : Inport  Port=9  OutDataType=sfix(16)  OutScaling=2^0
  in2_1 : Inport  Port=10  OutDataType=sfix(16)  OutScaling=2^0
  in2_2 : Inport  Port=11  OutDataType=sfix(16)  OutScaling=2^0
  in2_3 : Inport  Port=12  OutDataType=sfix(16)  OutScaling=2^0
  in2_4 : Inport  Port=13  OutDataType=sfix(16)  OutScaling=2^0
  in2_5 : Inport  Port=14  OutDataType=sfix(16)  OutScaling=2^0
  in2_6 : Inport  Port=15  OutDataType=sfix(16)  OutScaling=2^0
  in2_7 : Inport  Port=16  OutDataType=sfix(16)  OutScaling=2^0
  rst : Inport  Port=17  OutDataType=sfix(16)  OutScaling=2^0
  en : Inport  Port=18  OutDataType=sfix(16)  OutScaling=2^0
  accumul-12 : xbsIndex_r4/Accumulator  operation=Add  n_bits=32  overflow=Saturate  scale=1  rst=on  hasbypass=off  en=on  dbl_ovrd=off  use_behavioral_HDL=on  implementation=Fabric  block_type=accum
      settings: infoedit="Adder or subtractor-based accumulator.   Output type and binary point position match the input.<P><P>Hardware notes: When \"Reinitialize with input 'b' on reset\" is selected, the accumulator is forced to run at the system rate even if the input 'b' is running at a slower rate."  xl_area=[0, 0, 0, 0, 0, 0, 0]  block_version=10.1.3
  add1_{1..4} (4 alike) : xbsIndex_r4/AddSub  mode=Addition  use_carryin=off  use_carryout=off  en=off  latency=1  precision=Full  arith_type=Unsigned  n_bits=8  bin_pt=2  quantization=Truncate  overflow=Wrap  dbl_ovrd=off  use_behavioral_HDL=on  pipelined=on  use_rpm=on  hw_selection=Fabric  block_type=addsub
      settings: xl_area=[0, 0, 0, 0, 0, 0, 0]  block_version=10.1.3
  add2_1 : xbsIndex_r4/AddSub  mode=Addition  use_carryin=off  use_carryout=off  en=off  latency=1  precision=Full  arith_type=Unsigned  n_bits=8  bin_pt=2  quantization=Truncate  overflow=Wrap  dbl_ovrd=off  use_behavioral_HDL=on  pipelined=on  use_rpm=on  hw_selection=Fabric  block_type=addsub
      settings: xl_area=[0, 0, 0, 0, 0, 0, 0]  block_version=10.1.3
  add2_2 : xbsIndex_r4/AddSub  mode=Addition  use_carryin=off  use_carryout=off  en=off  latency=1  precision=Full  arith_type=Unsigned  n_bits=8  bin_pt=2  quantization=Truncate  overflow=Wrap  dbl_ovrd=off  use_behavioral_HDL=on  pipelined=on  use_rpm=on  hw_selection=Fabric  block_type=addsub
      settings: xl_area=[0, 0, 0, 0, 0, 0, 0]  block_version=10.1.3
  add3_1 : xbsIndex_r4/AddSub  mode=Addition  use_carryin=off  use_carryout=off  en=off  latency=1  precision=Full  arith_type=Unsigned  n_bits=8  bin_pt=2  quantization=Truncate  overflow=Wrap  dbl_ovrd=off  use_behavioral_HDL=on  pipelined=on  use_rpm=on  hw_selection=Fabric  block_type=addsub
      settings: xl_area=[0, 0, 0, 0, 0, 0, 0]  block_version=10.1.3
  mult{1..8} (8 alike) : quant_xcorr_lib/2bit_mult  SystemSampleTime=-1
  acc_out : Outport  OutDataType=sfix(16)  OutScaling=2^0
  wires:
  in1_0/1 -> mult1/1
  in2_0/1 -> mult1/2
  in1_1/1 -> mult2/1
  in2_1/1 -> mult2/2
  in1_2/1 -> mult3/1
  in2_2/1 -> mult3/2
  in1_3/1 -> mult4/1
  in2_3/1 -> mult4/2
  in1_4/1 -> mult5/1
  in2_4/1 -> mult5/2
  in1_5/1 -> mult6/1
  in2_5/1 -> mult6/2
  in1_6/1 -> mult7/1
  in2_6/1 -> mult7/2
  in1_7/1 -> mult8/1
  in2_7/1 -> mult8/2
  mult1/1 -> add1_1/1
  mult2/1 -> add1_1/2
  mult3/1 -> add1_2/1
  mult4/1 -> add1_2/2
  mult5/1 -> add1_3/1
  mult6/1 -> add1_3/2
  mult7/1 -> add1_4/1
  mult8/1 -> add1_4/2
  add1_1/1 -> add2_1/1
  add1_2/1 -> add2_1/2
  add1_3/1 -> add2_2/1
  add1_4/1 -> add2_2/2
  add2_1/1 -> add3_1/1
  add2_2/1 -> add3_1/2
  add3_1/1 -> accumul-12/1
  accumul-12/1 -> acc_out/1
  rst/1 -> accumul-12/2
  en/1 -> accumul-12/3

== system bee2_calib_corr/quant_cross_corr0/lag-13 ==
  in1_0 : Inport  OutDataType=sfix(16)  OutScaling=2^0
  in1_1 : Inport  Port=2  OutDataType=sfix(16)  OutScaling=2^0
  in1_2 : Inport  Port=3  OutDataType=sfix(16)  OutScaling=2^0
  in1_3 : Inport  Port=4  OutDataType=sfix(16)  OutScaling=2^0
  in1_4 : Inport  Port=5  OutDataType=sfix(16)  OutScaling=2^0
  in1_5 : Inport  Port=6  OutDataType=sfix(16)  OutScaling=2^0
  in1_6 : Inport  Port=7  OutDataType=sfix(16)  OutScaling=2^0
  in1_7 : Inport  Port=8  OutDataType=sfix(16)  OutScaling=2^0
  in2_0 : Inport  Port=9  OutDataType=sfix(16)  OutScaling=2^0
  in2_1 : Inport  Port=10  OutDataType=sfix(16)  OutScaling=2^0
  in2_2 : Inport  Port=11  OutDataType=sfix(16)  OutScaling=2^0
  in2_3 : Inport  Port=12  OutDataType=sfix(16)  OutScaling=2^0
  in2_4 : Inport  Port=13  OutDataType=sfix(16)  OutScaling=2^0
  in2_5 : Inport  Port=14  OutDataType=sfix(16)  OutScaling=2^0
  in2_6 : Inport  Port=15  OutDataType=sfix(16)  OutScaling=2^0
  in2_7 : Inport  Port=16  OutDataType=sfix(16)  OutScaling=2^0
  rst : Inport  Port=17  OutDataType=sfix(16)  OutScaling=2^0
  en : Inport  Port=18  OutDataType=sfix(16)  OutScaling=2^0
  accumul-13 : xbsIndex_r4/Accumulator  operation=Add  n_bits=32  overflow=Saturate  scale=1  rst=on  hasbypass=off  en=on  dbl_ovrd=off  use_behavioral_HDL=on  implementation=Fabric  block_type=accum
      settings: infoedit="Adder or subtractor-based accumulator.   Output type and binary point position match the input.<P><P>Hardware notes: When \"Reinitialize with input 'b' on reset\" is selected, the accumulator is forced to run at the system rate even if the input 'b' is running at a slower rate."  xl_area=[0, 0, 0, 0, 0, 0, 0]  block_version=10.1.3
  add1_{1..4} (4 alike) : xbsIndex_r4/AddSub  mode=Addition  use_carryin=off  use_carryout=off  en=off  latency=1  precision=Full  arith_type=Unsigned  n_bits=8  bin_pt=2  quantization=Truncate  overflow=Wrap  dbl_ovrd=off  use_behavioral_HDL=on  pipelined=on  use_rpm=on  hw_selection=Fabric  block_type=addsub
      settings: xl_area=[0, 0, 0, 0, 0, 0, 0]  block_version=10.1.3
  add2_1 : xbsIndex_r4/AddSub  mode=Addition  use_carryin=off  use_carryout=off  en=off  latency=1  precision=Full  arith_type=Unsigned  n_bits=8  bin_pt=2  quantization=Truncate  overflow=Wrap  dbl_ovrd=off  use_behavioral_HDL=on  pipelined=on  use_rpm=on  hw_selection=Fabric  block_type=addsub
      settings: xl_area=[0, 0, 0, 0, 0, 0, 0]  block_version=10.1.3
  add2_2 : xbsIndex_r4/AddSub  mode=Addition  use_carryin=off  use_carryout=off  en=off  latency=1  precision=Full  arith_type=Unsigned  n_bits=8  bin_pt=2  quantization=Truncate  overflow=Wrap  dbl_ovrd=off  use_behavioral_HDL=on  pipelined=on  use_rpm=on  hw_selection=Fabric  block_type=addsub
      settings: xl_area=[0, 0, 0, 0, 0, 0, 0]  block_version=10.1.3
  add3_1 : xbsIndex_r4/AddSub  mode=Addition  use_carryin=off  use_carryout=off  en=off  latency=1  precision=Full  arith_type=Unsigned  n_bits=8  bin_pt=2  quantization=Truncate  overflow=Wrap  dbl_ovrd=off  use_behavioral_HDL=on  pipelined=on  use_rpm=on  hw_selection=Fabric  block_type=addsub
      settings: xl_area=[0, 0, 0, 0, 0, 0, 0]  block_version=10.1.3
  mult{1..8} (8 alike) : quant_xcorr_lib/2bit_mult  SystemSampleTime=-1
  acc_out : Outport  OutDataType=sfix(16)  OutScaling=2^0
  wires:
  in1_0/1 -> mult1/1
  in2_0/1 -> mult1/2
  in1_1/1 -> mult2/1
  in2_1/1 -> mult2/2
  in1_2/1 -> mult3/1
  in2_2/1 -> mult3/2
  in1_3/1 -> mult4/1
  in2_3/1 -> mult4/2
  in1_4/1 -> mult5/1
  in2_4/1 -> mult5/2
  in1_5/1 -> mult6/1
  in2_5/1 -> mult6/2
  in1_6/1 -> mult7/1
  in2_6/1 -> mult7/2
  in1_7/1 -> mult8/1
  in2_7/1 -> mult8/2
  mult1/1 -> add1_1/1
  mult2/1 -> add1_1/2
  mult3/1 -> add1_2/1
  mult4/1 -> add1_2/2
  mult5/1 -> add1_3/1
  mult6/1 -> add1_3/2
  mult7/1 -> add1_4/1
  mult8/1 -> add1_4/2
  add1_1/1 -> add2_1/1
  add1_2/1 -> add2_1/2
  add1_3/1 -> add2_2/1
  add1_4/1 -> add2_2/2
  add2_1/1 -> add3_1/1
  add2_2/1 -> add3_1/2
  add3_1/1 -> accumul-13/1
  accumul-13/1 -> acc_out/1
  rst/1 -> accumul-13/2
  en/1 -> accumul-13/3

== system bee2_calib_corr/quant_cross_corr0/lag-14 ==
  in1_0 : Inport  OutDataType=sfix(16)  OutScaling=2^0
  in1_1 : Inport  Port=2  OutDataType=sfix(16)  OutScaling=2^0
  in1_2 : Inport  Port=3  OutDataType=sfix(16)  OutScaling=2^0
  in1_3 : Inport  Port=4  OutDataType=sfix(16)  OutScaling=2^0
  in1_4 : Inport  Port=5  OutDataType=sfix(16)  OutScaling=2^0
  in1_5 : Inport  Port=6  OutDataType=sfix(16)  OutScaling=2^0
  in1_6 : Inport  Port=7  OutDataType=sfix(16)  OutScaling=2^0
  in1_7 : Inport  Port=8  OutDataType=sfix(16)  OutScaling=2^0
  in2_0 : Inport  Port=9  OutDataType=sfix(16)  OutScaling=2^0
  in2_1 : Inport  Port=10  OutDataType=sfix(16)  OutScaling=2^0
  in2_2 : Inport  Port=11  OutDataType=sfix(16)  OutScaling=2^0
  in2_3 : Inport  Port=12  OutDataType=sfix(16)  OutScaling=2^0
  in2_4 : Inport  Port=13  OutDataType=sfix(16)  OutScaling=2^0
  in2_5 : Inport  Port=14  OutDataType=sfix(16)  OutScaling=2^0
  in2_6 : Inport  Port=15  OutDataType=sfix(16)  OutScaling=2^0
  in2_7 : Inport  Port=16  OutDataType=sfix(16)  OutScaling=2^0
  rst : Inport  Port=17  OutDataType=sfix(16)  OutScaling=2^0
  en : Inport  Port=18  OutDataType=sfix(16)  OutScaling=2^0
  accumul-14 : xbsIndex_r4/Accumulator  operation=Add  n_bits=32  overflow=Saturate  scale=1  rst=on  hasbypass=off  en=on  dbl_ovrd=off  use_behavioral_HDL=on  implementation=Fabric  block_type=accum
      settings: infoedit="Adder or subtractor-based accumulator.   Output type and binary point position match the input.<P><P>Hardware notes: When \"Reinitialize with input 'b' on reset\" is selected, the accumulator is forced to run at the system rate even if the input 'b' is running at a slower rate."  xl_area=[0, 0, 0, 0, 0, 0, 0]  block_version=10.1.3
  add1_{1..4} (4 alike) : xbsIndex_r4/AddSub  mode=Addition  use_carryin=off  use_carryout=off  en=off  latency=1  precision=Full  arith_type=Unsigned  n_bits=8  bin_pt=2  quantization=Truncate  overflow=Wrap  dbl_ovrd=off  use_behavioral_HDL=on  pipelined=on  use_rpm=on  hw_selection=Fabric  block_type=addsub
      settings: xl_area=[0, 0, 0, 0, 0, 0, 0]  block_version=10.1.3
  add2_1 : xbsIndex_r4/AddSub  mode=Addition  use_carryin=off  use_carryout=off  en=off  latency=1  precision=Full  arith_type=Unsigned  n_bits=8  bin_pt=2  quantization=Truncate  overflow=Wrap  dbl_ovrd=off  use_behavioral_HDL=on  pipelined=on  use_rpm=on  hw_selection=Fabric  block_type=addsub
      settings: xl_area=[0, 0, 0, 0, 0, 0, 0]  block_version=10.1.3
  add2_2 : xbsIndex_r4/AddSub  mode=Addition  use_carryin=off  use_carryout=off  en=off  latency=1  precision=Full  arith_type=Unsigned  n_bits=8  bin_pt=2  quantization=Truncate  overflow=Wrap  dbl_ovrd=off  use_behavioral_HDL=on  pipelined=on  use_rpm=on  hw_selection=Fabric  block_type=addsub
      settings: xl_area=[0, 0, 0, 0, 0, 0, 0]  block_version=10.1.3
  add3_1 : xbsIndex_r4/AddSub  mode=Addition  use_carryin=off  use_carryout=off  en=off  latency=1  precision=Full  arith_type=Unsigned  n_bits=8  bin_pt=2  quantization=Truncate  overflow=Wrap  dbl_ovrd=off  use_behavioral_HDL=on  pipelined=on  use_rpm=on  hw_selection=Fabric  block_type=addsub
      settings: xl_area=[0, 0, 0, 0, 0, 0, 0]  block_version=10.1.3
  mult{1..8} (8 alike) : quant_xcorr_lib/2bit_mult  SystemSampleTime=-1
  acc_out : Outport  OutDataType=sfix(16)  OutScaling=2^0
  wires:
  in1_0/1 -> mult1/1
  in2_0/1 -> mult1/2
  in1_1/1 -> mult2/1
  in2_1/1 -> mult2/2
  in1_2/1 -> mult3/1
  in2_2/1 -> mult3/2
  in1_3/1 -> mult4/1
  in2_3/1 -> mult4/2
  in1_4/1 -> mult5/1
  in2_4/1 -> mult5/2
  in1_5/1 -> mult6/1
  in2_5/1 -> mult6/2
  in1_6/1 -> mult7/1
  in2_6/1 -> mult7/2
  in1_7/1 -> mult8/1
  in2_7/1 -> mult8/2
  mult1/1 -> add1_1/1
  mult2/1 -> add1_1/2
  mult3/1 -> add1_2/1
  mult4/1 -> add1_2/2
  mult5/1 -> add1_3/1
  mult6/1 -> add1_3/2
  mult7/1 -> add1_4/1
  mult8/1 -> add1_4/2
  add1_1/1 -> add2_1/1
  add1_2/1 -> add2_1/2
  add1_3/1 -> add2_2/1
  add1_4/1 -> add2_2/2
  add2_1/1 -> add3_1/1
  add2_2/1 -> add3_1/2
  add3_1/1 -> accumul-14/1
  accumul-14/1 -> acc_out/1
  rst/1 -> accumul-14/2
  en/1 -> accumul-14/3
